simulink model "TosAeb"  (R2021a)
settings: Solver=FixedStepDiscrete  StartTime=0.0  StopTime=inf  FixedStep=0.01  RelTol=1e-3
undefined symbols (not defined in the file or in its folder): ACCModSts, AccSts1, AEB_ObjectMovingOrientation, AEB_ObjectMovingState, CN_BTCActive, CN_CurrentGearR, CN_CurrentGearR_DHT, CN_PTCActive, CN_VDCActive, CN_VSC_EspOff, CN_VSC_FCW_AEB_Error, CN_VSC_FCW_AEB_NoDispay, CN_VSC_FCW_AEB_PerfmDgdtn, CN_VSC_FCW_AEB_TempUnAvlbl, CN_VSC_VehStandstill, CNaccelAvoidMax, CNaccelAvoidMin, CNbrakePedalPress, CNcomfortStrLeftAccelMax, CNcomfortStrLeftAccelMin, CNcomfortStrRightAccelMax, CNcomfortStrRightAccelMin, CNDrvDoorOpen, CNDrvModeEco, CNDrvModeNorm, CNDrvModeOther, CNDrvModeSport, CNDrvSBRreminder, CNNull, CNobjInvalidID, CNPassDoorOpen, CNPassSBRreminder, CNPEBSAccelNegInf, CNPEBSAccelNull, CNPEBSTimeInf, CNPEBSTimeNull, CNSTOPMAX, CNSTOPNULL, CNsubjWidth, CNSwtOFF, CNSwtON, CNTTCMAX, CNTTCNULL, CNVehConfigAEBCycNone, CNVehConfigAEBNone, CNVehConfigAEBPedNone, CNVehConfigFCWNone, CNVehConfigJANone, CollisionSeverity, FALSE, FCWSnvty, JACycObjCalcInfoDefaultParams, JAPedObjCalcInfoDefaultParams, JAVehObjCalcInfoDefaultParams, latCycObjCalcInfoDefaultParams, latPedObjCalcInfoDefaultParams, longObjCalcInfoDefaultParams, ModSts, movingStatus, ObjectType ... (+1112)
libraries: simulink x3031, AEBSFunctionLib x50, Ta_Library x33, AEBSStateMachineLib x23, Mylibrary x12, simulink_extras x7, ObjSelect_lib x3, dspstat3 x2
(part 1 of 102)
settings Model: DataDictionary=ADAS.sldd  Creator=jiapengfei  LastModifiedBy=jiapengfei
settings OptimizationCC: DefaultParameterBehavior=Inlined  EnhancedBackFolding=on  PassReuseOutputArgsAs=Individual arguments  ZeroExternalMemoryAtStartup=off  ZeroInternalMemoryAtStartup=off  OptimizeBlockOrder=speed  DifferentSizesBufferReuse=on  OptimizationCustomize=off  EfficientTunableParamExpr=on
settings DebuggingCC: ParameterTunabilityLossMsg=error
settings SFSimCC: MATLABDynamicMemAlloc=off
settings RTWCC: SystemTargetFile=ert.tlc  GenCodeOnly=on  TemplateMakefile=ert_default_tmf  GenerateReport=on  Toolchain=Microsoft Visual C++ 2019 v16.0 | nmake (64-bit Windows)  BuildConfiguration=Debug  IncludeHyperlinkInReport=on  LaunchReport=on  PortableWordSizes=on  GenerateTraceInfo=on  GenerateTraceReport=on  GenerateTraceReportSl=on  GenerateTraceReportSf=on  GenerateTraceReportEml=on  GenerateCodeMetricsReport=on
settings CodeAppCC: ForceParamTrailComments=on  IgnoreCustomStorageClasses=off  MaxIdLength=128  OperatorAnnotations=on  SimulinkDataObjDesc=on  SFDataObjDesc=on  InsertBlockDesc=on
settings ERTTargetCC: TargetLangStandard=C99 (ISO)  GenerateSampleERTMain=on  InlinedParameterPlacement=NonHierarchical  ERTCustomFileTemplate=example_file_process.tlc  ERTFilePackagingFormat=Compact

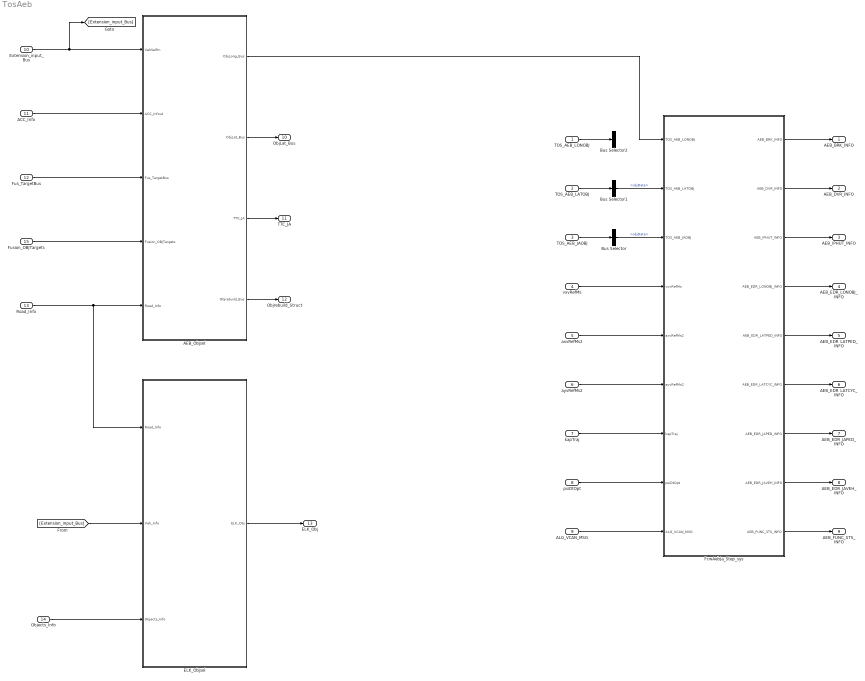
== system TosAeb ==
  TOS_AEB_LONOBJ : Inport  OutDataTypeStr=Bus: ObjectAEBLon_Struct  PortDimensions=1  BusOutputAsStruct=on
  TOS_AEB_LATOBJ : Inport  Port=2  OutDataTypeStr=Bus: ObjectAEBLat_Struct  PortDimensions=1  BusOutputAsStruct=on
  TOS_AEB_JAOBJ : Inport  Port=3  OutDataTypeStr=Bus: Objects_Struct  PortDimensions=1  BusOutputAsStruct=on
  vxvRefMs : Inport  Port=4  OutDataTypeStr=single  PortDimensions=1  BusOutputAsStruct=on
  axvRefMs2 : Inport  Port=5  OutDataTypeStr=single  PortDimensions=1  BusOutputAsStruct=on
  ayvRefMs2 : Inport  Port=6  OutDataTypeStr=single  PortDimensions=1  BusOutputAsStruct=on
  kapTraj : Inport  Port=7  OutDataTypeStr=single  PortDimensions=1  BusOutputAsStruct=on
  psiDtOpt : Inport  Port=8  OutDataTypeStr=single  PortDimensions=1  BusOutputAsStruct=on
  ALG_VCAN_MSG : Inport  Port=9  OutDataTypeStr=Bus: VehicleInfo_Struct  PortDimensions=1  BusOutputAsStruct=on
  Extension_input_Bus : Inport  Port=10  OutDataTypeStr=Bus: Extension_input_Bus  BusOutputAsStruct=on
  ACC_Info : Inport  Port=11  OutDataTypeStr=Bus: ACC_Infout  BusOutputAsStruct=on
  Fus_TargetBus : Inport  Port=12  OutDataTypeStr=Bus: TA_ObjProInfo  BusOutputAsStruct=on
  Road_Info : Inport  Port=13  OutDataTypeStr=Bus: Road_struct  BusOutputAsStruct=on
  Objects_Info : Inport  Port=14  OutDataTypeStr=Bus: Objects_Struct  BusOutputAsStruct=on
  Fusion_OBJTargets : Inport  Port=15  OutDataTypeStr=Bus: Objects_Struct  PortDimensions=1  BusOutputAsStruct=on
  AEB_Objsel : Subsystem
  Bus Selector : BusSelector  OutputSignals=objData
  Bus Selector1 : BusSelector  OutputSignals=objData
  Bus Selector2 : BusSelector  OutputSignals=objData
  ELK_Objsel : Subsystem
  FcwAebJa_Step_sys : Subsystem  TreatAsAtomicUnit=on  SystemSampleTime=0.02
  AEB_BRK_INFO : Outport  OutDataTypeStr=Bus: AEB_BRK_INFO_BUS  PortDimensions=1  BusOutputAsStruct=on
  AEB_DVR_INFO : Outport  Port=2  OutDataTypeStr=Bus: AEB_DVR_INFO_BUS  PortDimensions=1  BusOutputAsStruct=on
  AEB_IPHUT_INFO : Outport  Port=3  OutDataTypeStr=Bus: AEB_IPHUT_INFO_BUS  PortDimensions=1  BusOutputAsStruct=on
  AEB_EDR_LONOBJ_INFO : Outport  Port=4  OutDataTypeStr=Bus: AEB_EDR_LONOBJ_INFO_BUS  PortDimensions=1  BusOutputAsStruct=on
  AEB_EDR_LATPED_INFO : Outport  Port=5  OutDataTypeStr=Bus: AEB_EDR_LATPED_INFO_BUS  PortDimensions=1  BusOutputAsStruct=on
  AEB_EDR_LATCYC_INFO : Outport  Port=6  OutDataTypeStr=Bus: AEB_EDR_LATCYC_INFO_BUS  PortDimensions=1  BusOutputAsStruct=on
  AEB_EDR_JAPED_INFO : Outport  Port=7  OutDataTypeStr=Bus: AEB_EDR_JAPED_INFO_BUS  PortDimensions=1  BusOutputAsStruct=on
  AEB_EDR_JAVEH_INFO : Outport  Port=8  OutDataTypeStr=Bus: AEB_EDR_JAVEH_INFO_BUS  PortDimensions=1  BusOutputAsStruct=on
  AEB_FUNC_STS_INFO : Outport  Port=9  OutDataTypeStr=Bus: AEB_FUNC_STS_INFO_BUS  PortDimensions=1  BusOutputAsStruct=on
  ObjLat_Bus : Outport  Port=10  OutDataTypeStr=Bus: objLat_Bus
  TTC_JA : Outport  Port=11
  Objrebuild_Struct : Outport  Port=12  OutDataTypeStr=Bus: Objrebuild_Bus
  ELK_Obj : Outport  Port=13  OutDataTypeStr=Bus: ObjectELK_Struct  BusOutputAsStruct=on
  wires:
  FcwAebJa_Step_sys/4(AEB_EDR_LONOBJ_INFO) -> AEB_EDR_LONOBJ_INFO/1
  FcwAebJa_Step_sys/7(AEB_EDR_JAPED_INFO) -> AEB_EDR_JAPED_INFO/1
  FcwAebJa_Step_sys/6(AEB_EDR_LATCYC_INFO) -> AEB_EDR_LATCYC_INFO/1
  FcwAebJa_Step_sys/3(AEB_IPHUT_INFO) -> AEB_IPHUT_INFO/1
  FcwAebJa_Step_sys/5(AEB_EDR_LATPED_INFO) -> AEB_EDR_LATPED_INFO/1
  FcwAebJa_Step_sys/2(AEB_DVR_INFO) -> AEB_DVR_INFO/1
  FcwAebJa_Step_sys/1(AEB_BRK_INFO) -> AEB_BRK_INFO/1
  FcwAebJa_Step_sys/9(AEB_FUNC_STS_INFO) -> AEB_FUNC_STS_INFO/1
  FcwAebJa_Step_sys/8(AEB_EDR_JAVEH_INFO) -> AEB_EDR_JAVEH_INFO/1
  TOS_AEB_JAOBJ/1 -> Bus Selector/1
  Bus Selector/1 -> FcwAebJa_Step_sys/3(TOS_AEB_JAOBJ)  "<objData>"
  TOS_AEB_LATOBJ/1 -> Bus Selector1/1
  Bus Selector1/1 -> FcwAebJa_Step_sys/2(TOS_AEB_LATOBJ)  "<objData>"
  TOS_AEB_LONOBJ/1 -> Bus Selector2/1
  psiDtOpt/1 -> FcwAebJa_Step_sys/8(psiDtOpt)
  ayvRefMs2/1 -> FcwAebJa_Step_sys/6(ayvRefMs2)
  kapTraj/1 -> FcwAebJa_Step_sys/7(kapTraj)
  vxvRefMs/1 -> FcwAebJa_Step_sys/4(vxvRefMs)
  axvRefMs2/1 -> FcwAebJa_Step_sys/5(axvRefMs2)
  ALG_VCAN_MSG/1 -> FcwAebJa_Step_sys/9(ALG_VCAN_MSG)
  AEB_Objsel/4(Objrebuild_Bus) -> Objrebuild_Struct/1
  AEB_Objsel/2(ObjLat_Bus) -> ObjLat_Bus/1
  ELK_Objsel/1(ELK_Obj) -> ELK_Obj/1
  AEB_Objsel/3(TTC_JA) -> TTC_JA/1
  Objects_Info/1 -> ELK_Objsel/3(Objects_Info)
  [Extension_input_Bus] -> ELK_Objsel/2(Veh_Info)
  Fus_TargetBus/1 -> AEB_Objsel/3(Fus_TargetBus)
  Road_Info/1 -> ELK_Objsel/1(Road_Info), AEB_Objsel/5(Road_Info)
  ACC_Info/1 -> AEB_Objsel/2(ACC_Infout)
  Extension_input_Bus/1 -> [Extension_input_Bus], AEB_Objsel/1(VehSelfIn)
  AEB_Objsel/1(ObjLong_Bus) -> FcwAebJa_Step_sys/1(TOS_AEB_LONOBJ)
  Fusion_OBJTargets/1 -> AEB_Objsel/4(Fusion_OBJTargets)

== system TosAeb/AEB_Objsel ==
  VehSelfIn : Inport
  ACC_Infout : Inport  Port=2
  Fus_TargetBus : Inport  Port=3
  Fusion_OBJTargets : Inport  Port=4  OutDataTypeStr=Bus: Objects_Struct  PortDimensions=1  BusOutputAsStruct=on
  Road_Info : Inport  Port=5
  Bus Creator : BusCreator  Commented=on  Inputs=3  OutDataTypeStr=Bus: objLat_Bus  NonVirtualBus=on
  Bus Selector1 : BusSelector  OutputSignals=ObjSortNum_Long
  Bus Selector2 : BusSelector  OutputSignals=ID_Lat
  Bus Selector3 : BusSelector  OutputSignals=ObjSortNum_Lat
  Bus Selector4 : BusSelector  OutputSignals=objData
  Constant : Constant
  For Iterator Subsystem : Subsystem  Commented=on  TreatAsAtomicUnit=on
  Lat_Check : MATLAB Function  Commented=on  TreatAsAtomicUnit=on
  Selector : Selector  InputPortWidth=32  IndexOptions=Starting index (port)  Indices=1  OutputSizes=1
  Signal Conversion : SignalConversion  Commented=on  OverrideOpt=off
  Signal Conversion1 : SignalConversion  Commented=on  OverrideOpt=off
  Signal Conversion2 : SignalConversion  Commented=on  OverrideOpt=off
  TA_InfoRebuild : Subsystem
  TA_Processor : Subsystem
  TOS_AEB_Lat_Attributes : Subsystem  TreatAsAtomicUnit=on
  ObjLong_Bus : Outport
  ObjLat_Bus : Outport  Port=2
  TTC_JA : Outport  Port=3
  Objrebuild_Bus : Outport  Port=4
  wires:
  VehSelfIn/1 -> TA_Processor/1(VehSelfIn)
  ACC_Infout/1 -> TA_Processor/2(ACC_Infout)
  TA_Processor/3(TTC_JA) -> TTC_JA/1
  Fus_TargetBus/1 -> TA_InfoRebuild/1(Fus_TargetBus), TA_Processor/3(Fus_TargetBus)
  TA_InfoRebuild/1(Objrebuild_Bus) -> Objrebuild_Bus/1
  Road_Info/1 -> TA_InfoRebuild/2(Road_Info)
  For Iterator Subsystem/1(ttc) -> Lat_Check/3(Lat_TTC)
  Lat_Check/2(Num) -> Signal Conversion/1
  Lat_Check/3(Ttc) -> Signal Conversion2/1
  Signal Conversion2/1 -> Bus Creator/3  "TTC_Lat"
  Signal Conversion/1 -> Bus Creator/2  "ObjSortNum_Lat"
  Bus Selector2/1 -> Lat_Check/1(Lat_ObjID)  "<ID_Lat>"
  Signal Conversion1/1 -> Bus Creator/1  "ID_Lat"
  Lat_Check/1(ID) -> Signal Conversion1/1
  TA_Processor/2(ObjLat_Bus) -> Bus Selector3/1, Bus Selector2/1, ObjLat_Bus/1
  Bus Selector3/1 -> Lat_Check/2(Lat_ObjSortNum), For Iterator Subsystem/1(Lat_ObjSortNum)  "<ObjSortNum_Lat>"
  TA_Processor/1(ObjLong_Bus) -> Bus Selector1/1
  Bus Selector1/1 -> Selector/2  "<ObjSortNum_Long>"
  Fusion_OBJTargets/1 -> Bus Selector4/1
  Bus Selector4/1 -> TOS_AEB_Lat_Attributes/2(TOS_PP_OFM_SignalVerification), For Iterator Subsystem/2(Lat_TTC), Selector/1  "<objData>"
  Selector/1 -> ObjLong_Bus/1
  Constant/1 -> TOS_AEB_Lat_Attributes/1(TOS_AEB_Lat_Index)

== system TosAeb/ELK_Objsel ==
  note: ELK Target Selection
  Road_Info : Inport  OutDataTypeStr=Bus: Road_struct
  Veh_Info : Inport  Port=2
  Objects_Info : Inport  Port=3  OutDataTypeStr=Bus: Objects_Struct
  Bus Creator : BusCreator  Inputs=4  OutDataTypeStr=Bus: ObjectELK_Struct  NonVirtualBus=on
  Bus Selector : BusSelector  OutputSignals=SAS_SteeringWheelSpeed,ABS_VehicleSpeed,SAS_SteeringWheelAngle,YawRate
  Bus Selector2 : BusSelector  OutputSignals=lineData
  Bus Selector3 : BusSelector  OutputSignals=objData
  Bus Selector4 : BusSelector  OutputSignals=timeStamp
  Constant : Constant  Value=1.95  OutDataTypeStr=single
  Constant1 : Constant  Value=0.02
  Constant2 : Constant  Value=3.9  OutDataTypeStr=single
  Constant3 : Constant  Value=4.9  OutDataTypeStr=single
  Constant4 : Constant  Value=0.001  OutDataTypeStr=single
  ELK_Left_Obj_Delay : Subsystem
  ELK_Left_Obj_Delay1 : Subsystem
  ELK_Pre_Cal : Subsystem
  EgoTrajCurvatureForFVSR : Subsystem
  Elk_ObjnumCal : Subsystem
  Info : Subsystem
      mask Display: fprintf('Put your\nimplementation\nhere')
  LanesSelect : Subsystem
  MATLAB Function : MATLAB Function  TreatAsAtomicUnit=on
  Select Object Info1 : Subsystem
  Select Object Info2 : Subsystem
  Signal Conversion{,1..4} (5 alike) : SignalConversion  OverrideOpt=off
  Target_Selection : Subsystem
  Terminator : Terminator
  ELK_Obj : Outport  OutDataTypeStr=Bus: ObjectELK_Struct
  wires:
  [right_boundary_lat_rate_filtered] -> Target_Selection/12(EgoTrajCurvature2)
  Target_Selection/1(dxObj_Vec) -> Terminator/1
  [SteerAngleSpd_degps] -> Target_Selection/8(SteerAngleSpd_degps)
  LanesSelect/1(LeftLineCoef) -> [LDCoef_L]  "LDCoef_L"
  [LDCoef_R] -> ELK_Pre_Cal/3(RightLine)
  Constant1/1 -> [Task_Period]
  [SteerAngle_Deg] -> Target_Selection/7(SteerAngle_Deg)
  ELK_Pre_Cal/2(right_boundary_lat_rate_filtered) -> [right_boundary_lat_rate_filtered]
  ELK_Left_Obj_Delay1/1(ELK_Right_Obj_out) -> [ELK_Right_Obj_out]
  [EgoTrajCurvature] -> Target_Selection/9(EgoTrajCurvature)
  [ELK_Left_Obj] -> ELK_Left_Obj_Delay/1(ELK_Left_Obj)
  [Index_Left_Obj] -> Select Object Info1/2(Index)
  Target_Selection/4(ELK_TA_Right_Flag_Vec) -> [ELK_TA_Right_Flag_Vec]
  [Host_Veh_Width] -> Target_Selection/3(Host_Veh_Width)
  [ELK_Right_Obj] -> ELK_Left_Obj_Delay1/1(ELK_Left_Obj)
  [VehSpdMps] -> EgoTrajCurvatureForFVSR/1(VehSpdMps)
  [ELK_RightLine_Valid_Flag] -> Target_Selection/14(EgoTrajCurvature4)
  ELK_Pre_Cal/1(left_boundary_lat_rate_filtered) -> [left_boundary_lat_rate_filtered]
  [Index_Right_Obj] -> Select Object Info2/2(Index)
  Target_Selection/2(dxObj_abs_Vec) -> [dxObj_abs_Vec]
  [Task_Period] -> ELK_Pre_Cal/4(Task_Period)
  [LDCoef_R] -> Target_Selection/16(LDCoef_R)
  Constant3/1 -> [Host_Veh_Length]
  [Objs_Obstacles] -> Target_Selection/1(Objs_Obstacles)
  [VehSpdMps] -> Target_Selection/5(VehSpdMps)
  [Host_Veh_Length] -> Target_Selection/2(Host_Veh_Length)
  [LDCoef_L] -> ELK_Pre_Cal/2(LeftLine)
  ELK_Pre_Cal/3(ELK_LeftLine_Valid_Flag) -> [ELK_LeftLine_Valid_Flag]
  ELK_Pre_Cal/4(ELK_RightLine_Valid_Flag) -> [ELK_RightLine_Valid_Flag]
  [left_boundary_lat_rate_filtered] -> Target_Selection/11(EgoTrajCurvature1)
  Target_Selection/3(ELK_TA_Left_Flag_Vec) -> [ELK_TA_Left_Flag_Vec]
  [dxDiffObjCoord2VehCoord] -> Target_Selection/4(dxDiffObjCoord2VehCoord)
  MATLAB Function/2(Index_Right_Obj) -> [Index_Right_Obj]  "Meas_Index_Right_Obj"
  [ELK_TA_Left_Flag_Vec] -> MATLAB Function/1(TA_Left_Flag_Vec)
  Select Object Info1/1(TargetInfo) -> [ELK_Left_Obj]
  Constant/1 -> [Host_Veh_Width]
  [Objs_Obstacles] -> Select Object Info1/1(Objs_all)
  LanesSelect/2(RightLineCoef) -> [LDCoef_R]  "LDCoef_R"
  [ELK_TA_Right_Flag_Vec] -> MATLAB Function/2(TA_Right_Flag_Vec)
  [Objs_Obstacles] -> Select Object Info2/1(Objs_all)
  ELK_Left_Obj_Delay/1(ELK_Left_Obj_out) -> [ELK_Left_Obj_out]
  Select Object Info2/1(TargetInfo) -> [ELK_Right_Obj]
  [LDCoef_L] -> Target_Selection/15(LDCoef_L)
  [dxObj_abs_Vec] -> MATLAB Function/3(dx_Obj_vector)
  EgoTrajCurvatureForFVSR/1(EgoTrajCurvatureForFVSR) -> [EgoTrajCurvatureForFVSR]
  [ELK_LeftLine_Valid_Flag] -> Target_Selection/13(EgoTrajCurvature3)
  [YawRate_Degps] -> Target_Selection/6(YawRate_Degps)
  [EgoTrajCurvature] -> EgoTrajCurvatureForFVSR/2(EgoTrajCurvature)
  [EgoTrajCurvatureForFVSR] -> Target_Selection/10(EgoTrajCurvatureForFVSR)
  MATLAB Function/1(Index_Left_Obj) -> [Index_Left_Obj]  "Meas_Index_Left_Obj"
  [Host_Veh_Width] -> ELK_Pre_Cal/1(Host_Veh_Width)
  Constant2/1 -> [dxDiffObjCoord2VehCoord]
  Veh_Info/1 -> Bus Selector/1
  Bus Selector/1 -> Signal Conversion3/1  "<SAS_SteeringWheelSpeed>"
  Bus Selector/2 -> Signal Conversion2/1  "<ABS_VehicleSpeed>"
  Bus Selector/3 -> Signal Conversion1/1  "<SAS_SteeringWheelAngle>"
  Bus Selector/4 -> Signal Conversion/1  "<YawRate>"
  Signal Conversion/1 -> [YawRate_Degps]
  Signal Conversion1/1 -> [SteerAngle_Deg]
  Signal Conversion2/1 -> [VehSpdMps]
  Signal Conversion3/1 -> [SteerAngleSpd_degps]
  Constant4/1 -> Signal Conversion4/1
  Signal Conversion4/1 -> [EgoTrajCurvature]
  Road_Info/1 -> Bus Selector2/1
  Bus Selector2/1 -> LanesSelect/1(lineData)  "<lineData>"
  Objects_Info/1 -> [allObject], Bus Selector3/1
  Bus Selector3/1 -> [Objs_Obstacles]  "<objData>"
  [ELK_Left_Obj_out] -> Elk_ObjnumCal/1(Left)
  [ELK_Right_Obj_out] -> Elk_ObjnumCal/2(Right)
  [allObject] -> Bus Selector4/1
  Bus Selector4/1 -> Bus Creator/1  "<timeStamp>"
  Elk_ObjnumCal/1(objnum) -> Bus Creator/2  "objNum"
  [ELK_Left_Obj_out] -> Bus Creator/3  "objELKLeft"
  [ELK_Right_Obj_out] -> Bus Creator/4  "objELKRight"
  Bus Creator/1 -> ELK_Obj/1

== system TosAeb/FcwAebJa_Step_sys ==
  TOS_AEB_LONOBJ : Inport  OutDataTypeStr=Bus: Object_Struct  PortDimensions=1  BusOutputAsStruct=on
  TOS_AEB_LATOBJ : Inport  Port=2  OutDataTypeStr=Bus: Object_Struct  PortDimensions=10  BusOutputAsStruct=on
  TOS_AEB_JAOBJ : Inport  Port=3  OutDataTypeStr=Bus: Object_Struct  PortDimensions=32  BusOutputAsStruct=on
  vxvRefMs : Inport  Port=4  OutDataTypeStr=single  PortDimensions=1  BusOutputAsStruct=on
  axvRefMs2 : Inport  Port=5  OutDataTypeStr=single  PortDimensions=1  BusOutputAsStruct=on
  ayvRefMs2 : Inport  Port=6  OutDataTypeStr=single  PortDimensions=1  BusOutputAsStruct=on
  kapTraj : Inport  Port=7  OutDataTypeStr=single  PortDimensions=1  BusOutputAsStruct=on
  psiDtOpt : Inport  Port=8  OutDataTypeStr=single  PortDimensions=1  BusOutputAsStruct=on
  ALG_VCAN_MSG : Inport  Port=9  OutDataTypeStr=Bus: VehicleInfo_Struct  PortDimensions=1  BusOutputAsStruct=on
  ALG_AEBSystem : Subsystem  TreatAsAtomicUnit=on
  AEB_BRK_INFO : Outport  OutDataTypeStr=Bus: AEB_BRK_INFO_BUS  PortDimensions=1  BusOutputAsStruct=on
  AEB_DVR_INFO : Outport  Port=2  OutDataTypeStr=Bus: AEB_DVR_INFO_BUS  PortDimensions=1  BusOutputAsStruct=on
  AEB_IPHUT_INFO : Outport  Port=3  OutDataTypeStr=Bus: AEB_IPHUT_INFO_BUS  PortDimensions=1  BusOutputAsStruct=on
  AEB_EDR_LONOBJ_INFO : Outport  Port=4  OutDataTypeStr=Bus: AEB_EDR_LONOBJ_INFO_BUS  PortDimensions=1  BusOutputAsStruct=on
  AEB_EDR_LATPED_INFO : Outport  Port=5  OutDataTypeStr=Bus: AEB_EDR_LATPED_INFO_BUS  PortDimensions=1  BusOutputAsStruct=on
  AEB_EDR_LATCYC_INFO : Outport  Port=6  OutDataTypeStr=Bus: AEB_EDR_LATCYC_INFO_BUS  PortDimensions=1  BusOutputAsStruct=on
  AEB_EDR_JAPED_INFO : Outport  Port=7  OutDataTypeStr=Bus: AEB_EDR_JAPED_INFO_BUS  PortDimensions=1  BusOutputAsStruct=on
  AEB_EDR_JAVEH_INFO : Outport  Port=8  OutDataTypeStr=Bus: AEB_EDR_JAVEH_INFO_BUS  PortDimensions=1  BusOutputAsStruct=on
  AEB_FUNC_STS_INFO : Outport  Port=9  OutDataTypeStr=Bus: AEB_FUNC_STS_INFO_BUS  PortDimensions=1  BusOutputAsStruct=on
  wires:
  ALG_AEBSystem/4(AEB_EDR_LONOBJ_INFO) -> AEB_EDR_LONOBJ_INFO/1
  ALG_AEBSystem/9(AEB_FUNC_STS_INFO) -> AEB_FUNC_STS_INFO/1
  ALG_AEBSystem/8(AEB_EDR_JAVEH_INFO) -> AEB_EDR_JAVEH_INFO/1
  ALG_AEBSystem/2(AEB_DVR_INFO) -> AEB_DVR_INFO/1
  ALG_AEBSystem/3(AEB_IPHUT_INFO) -> AEB_IPHUT_INFO/1
  ALG_AEBSystem/7(AEB_EDR_JAPED_INFO) -> AEB_EDR_JAPED_INFO/1
  ALG_AEBSystem/1(AEB_BRK_INFO) -> AEB_BRK_INFO/1
  ALG_AEBSystem/5(AEB_EDR_LATPED_INFO) -> AEB_EDR_LATPED_INFO/1
  ALG_AEBSystem/6(AEB_EDR_LATCYC_INFO) -> AEB_EDR_LATCYC_INFO/1
  TOS_AEB_LONOBJ/1 -> ALG_AEBSystem/1(TOS_AEB_LONOBJ)
  TOS_AEB_LATOBJ/1 -> ALG_AEBSystem/2(TOS_AEB_LATOBJ)
  TOS_AEB_JAOBJ/1 -> ALG_AEBSystem/3(TOS_AEB_JAOBJ)
  vxvRefMs/1 -> ALG_AEBSystem/4(vxvRefMs)
  axvRefMs2/1 -> ALG_AEBSystem/5(axvRefMs2)
  ayvRefMs2/1 -> ALG_AEBSystem/6(ayvRefMs2)
  kapTraj/1 -> ALG_AEBSystem/7(kapTraj)
  psiDtOpt/1 -> ALG_AEBSystem/8(psiDtOpt)
  ALG_VCAN_MSG/1 -> ALG_AEBSystem/9(ALG_VCAN_MSG)

== system TosAeb/AEB_Objsel/For Iterator Subsystem ==
  Lat_ObjSortNum : Inport
  Lat_TTC : Inport  Port=2
  Assignment : Assignment  DiagnosticForDimensions=Warning  IndexOptions=Index vector (port)  Indices=1  OutputSizes=1
  Compare To Constant : simulink/Logic and Bit Operations/Compare To Constant  relop===  const=0  OutDataTypeStr=boolean
  Constant : Constant  Value=zeros(10,1)  OutDataTypeStr=single
  Constant1 : Constant  Value=100  OutDataTypeStr=single
  Constant2 : Constant  OutDataTypeStr=uint8
  For Iterator : ForIterator  IterationLimit=10
  Index Vector : MultiPortSwitch  Inputs=1
  Index Vector1 : MultiPortSwitch  Inputs=1
  Switch : Switch  Criteria=u2 > Threshold
  Switch1 : Switch  Criteria=u2 > Threshold
  ttc : Outport
  wires:
  Constant/1 -> Assignment/1
  Assignment/1 -> ttc/1
  For Iterator/1 -> [Index]
  [Index] -> Assignment/3
  [Index] -> Index Vector1/1
  Lat_ObjSortNum/1 -> Index Vector1/2
  Index Vector1/1 -> Switch1/3, Compare To Constant/1
  Compare To Constant/1 -> Switch1/2, Switch/2
  Constant1/1 -> Switch/1
  Constant2/1 -> Switch1/1
  Switch1/1 -> Index Vector/1
  Lat_TTC/1 -> Index Vector/2
  Switch/1 -> Assignment/2
  Index Vector/1 -> Switch/3

== system TosAeb/AEB_Objsel/TA_InfoRebuild ==
  Fus_TargetBus : Inport
  Road_Info : Inport  Port=2
  TAObjInfoCalc : Subsystem  TreatAsAtomicUnit=on
  Objrebuild_Bus : Outport
  wires:
  Fus_TargetBus/1 -> TAObjInfoCalc/1(Fus_TargetBus)
  Road_Info/1 -> TAObjInfoCalc/2(Road_Info)
  TAObjInfoCalc/1(Objrebuild_Struct) -> Objrebuild_Bus/1

== system TosAeb/AEB_Objsel/TA_Processor ==
  VehSelfIn : Inport
  ACC_Infout : Inport  Port=2
  Fus_TargetBus : Inport  Port=3
  AEB_ObjSelect : Subsystem
  PreProcessor : Subsystem
  TA_Check : Subsystem
  ObjLong_Bus : Outport
  ObjLat_Bus : Outport  Port=2
  TTC_JA : Outport  Port=3
  wires:
  VehSelfIn/1 -> PreProcessor/1(VehSelfIn)  "VehSelf"
  Fus_TargetBus/1 -> PreProcessor/3(Fus_TargetBus)
  PreProcessor/4(AccTgtIdn) -> AEB_ObjSelect/4(AccTgtIdn)
  PreProcessor/1(VehSelfData) -> AEB_ObjSelect/1(VehSelfData)
  ACC_Infout/1 -> PreProcessor/2(ACC_Infout)
  AEB_ObjSelect/1(Long_ObjSortNum) -> TA_Check/1(Long_ObjSortNum)
  PreProcessor/2(TA_ObjSumInfo) -> AEB_ObjSelect/2(TA_ObjSumInfo)
  PreProcessor/3(PathEstimn) -> AEB_ObjSelect/3(PathEstimn)
  AEB_ObjSelect/7(Lat_TTC) -> TA_Check/7(Lat_TTC)
  AEB_ObjSelect/2(Long_ObjID) -> TA_Check/2(Long_ObjID)
  AEB_ObjSelect/5(Ta_Info) -> TA_Check/5(Ta_Info)
  AEB_ObjSelect/3(Lat_ObjSortNum) -> TA_Check/3(Lat_ObjSortNum)
  AEB_ObjSelect/4(Lat_ObjID) -> TA_Check/4(Lat_ObjID)
  AEB_ObjSelect/6(Long_TTC) -> TA_Check/6(Long_TTC)
  AEB_ObjSelect/8(JA_TTC) -> TA_Check/8(JA_TTC)
  TA_Check/1(ObjLong_Bus) -> ObjLong_Bus/1
  TA_Check/2(ObjLat_Bus) -> ObjLat_Bus/1
  TA_Check/3(TTC_JA) -> TTC_JA/1

== system TosAeb/AEB_Objsel/TOS_AEB_Lat_Attributes ==
  TOS_AEB_Lat_Index : Inport  Partition=on  PartitionDimension=1  PartitionWidth=1  PartitionOffset=0
  TOS_PP_OFM_SignalVerification : Inport  Port=2
  Compare To Constant : simulink/Logic and Bit Operations/Compare To Constant  relop=~=  const=0  OutDataTypeStr=boolean
  For Each : ForEach  DisableCoverage=on
  If : If  IfExpression=u1 == 0
  If Action Subsystem : Subsystem  TreatAsAtomicUnit=on
  If Action Subsystem1 : Subsystem  TreatAsAtomicUnit=on
  Merge : Merge
  wires:
  TOS_AEB_Lat_Index/1 -> [TOS_AEB_Lat_Index], Compare To Constant/1
  Compare To Constant/1 -> If/1
  If/1 -> If Action Subsystem/Action
  TOS_PP_OFM_SignalVerification/1 -> If Action Subsystem1/2(TOS_PP_OFM_SignalVerification)
  If/2 -> If Action Subsystem1/Action
  [TOS_AEB_Lat_Index] -> If Action Subsystem1/1(TOS_AEB_Lat_Index)
  If Action Subsystem/1(Out1) -> Merge/1
  If Action Subsystem1/1(Out1) -> Merge/2

== system TosAeb/ELK_Objsel/ELK_Left_Obj_Delay ==
  ELK_Left_Obj : Inport
  Bus Creator : BusCreator  Commented=on  Inputs=3  OutDataTypeStr=Bus: ObjectELK_Struct  NonVirtualBus=on
  Bus Selector2 : BusSelector  OutputSignals=objID
  Compare To Constant : simulink/Logic and Bit Operations/Compare To Constant  Commented=on  relop===  const=0  OutDataTypeStr=boolean
  Constant : Constant  Commented=on  Value=50
  Constant1 : Constant  Commented=on  Value=0
  Constant10 : Constant  Value=0
  Constant11 : Constant  Value=0
  Constant2 : Constant  Commented=on  Value=0
  Constant3 : Constant  Commented=on  OutDataTypeStr=int64_t
  Constant4 : Constant  Commented=on  OutDataTypeStr=uint8_t
  Constant5 : Constant  Commented=on  Value=0  OutDataTypeStr=uint8_t
  Constant9 : Constant  Value=0
  From1 : From  Commented=on  GotoTag=ELK_Left_Obj_ID
  From51 : From  Commented=on  GotoTag=ELK_Left_Obj_ID
  Hysteresis2 : Stateflow chart  Commented=on  TreatAsAtomicUnit=on
  Relational Operator : RelationalOperator  Commented=on  Operator=~=  OutDataTypeStr=boolean  RndMeth=Simplest
  Relational Operator10 : RelationalOperator  Operator=>  OutDataTypeStr=boolean  RndMeth=Simplest
  Relational Operator7 : RelationalOperator  Commented=on  Operator===  OutDataTypeStr=boolean  RndMeth=Simplest
  Relational Operator8 : RelationalOperator  Operator=>  OutDataTypeStr=boolean  RndMeth=Simplest
  Relational Operator9 : RelationalOperator  Operator=>  OutDataTypeStr=boolean  RndMeth=Simplest
  Switch{,1..3} (4 alike) : Switch  Criteria=u2 > Threshold
  Switch4 : Switch  Commented=on  Criteria=u2 > Threshold
  Trigger Hold1 : Subsystem  Commented=on
  Unit Delay{,1,4..7} (6 alike) : UnitDelay  SampleTime=-1  HasFrameUpgradeWarning=on
  ELK_Left_Obj_out : Outport  OutDataTypeStr=Bus: Object_Struct
  wires:
  Trigger Hold1/1(Out) -> ?
  Unit Delay1/1 -> [ELK_Left_Obj_K1], Unit Delay4/1
  Unit Delay/1 -> Unit Delay6/1, [ELK_Left_Obj_ID_K1]
  [ELK_Left_Obj] -> Unit Delay1/1
  [ELK_Left_Obj_ID_K1] -> Relational Operator9/1
  Constant10/1 -> Relational Operator9/2
  Switch/1 -> [ELK_Left_Obj_out]
  [ELK_Left_Obj] -> Switch/1
  Constant2/1 -> Relational Operator7/2
  Hysteresis2/1(Flag) -> Trigger Hold1/1(Trigger)
  Constant/1 -> Trigger Hold1/2(hold_time)
  Relational Operator/1 -> Hysteresis2/1(Input_1)
  From51/1 -> Relational Operator7/1, Relational Operator/1
  Relational Operator7/1 -> Hysteresis2/2(Input_2)
  [ELK_Left_Obj] -> Bus Selector2/1
  Constant1/1 -> Relational Operator/2
  [ELK_Left_Obj_ID] -> Switch/2
  Unit Delay4/1 -> Unit Delay5/1, [ELK_Left_Obj_K2]
  Relational Operator9/1 -> Switch1/2
  Unit Delay7/1 -> [ELK_Left_Obj_ID_K3]
  Unit Delay5/1 -> [ELK_Left_Obj_K3]
  Unit Delay6/1 -> Unit Delay7/1, [ELK_Left_Obj_ID_K2]
  Switch2/1 -> Switch1/3
  Switch1/1 -> Switch/3
  [ELK_Left_Obj_K2] -> Switch2/1
  [ELK_Left_Obj] -> Switch3/3
  [ELK_Left_Obj_K1] -> Switch1/1
  [ELK_Left_Obj_ID_K2] -> Relational Operator8/1
  Constant9/1 -> Relational Operator8/2
  Relational Operator8/1 -> Switch2/2
  [ELK_Left_Obj_K3] -> Switch3/1
  [ELK_Left_Obj_ID_K3] -> Relational Operator10/1
  Constant11/1 -> Relational Operator10/2
  Relational Operator10/1 -> Switch3/2
  Switch3/1 -> Switch2/3
  Bus Selector2/1 -> Unit Delay/1, [ELK_Left_Obj_ID]  "<objID>"
  ELK_Left_Obj/1 -> [ELK_Left_Obj]
  From1/1 -> Compare To Constant/1
  Compare To Constant/1 -> Switch4/2
  Constant4/1 -> Switch4/1
  Constant5/1 -> Switch4/3
  Constant3/1 -> Bus Creator/1  "timeStamp"
  Switch4/1 -> Bus Creator/2  "objNum"
  Bus Creator/1 -> ?
  [ELK_Left_Obj_out] -> ELK_Left_Obj_out/1  "objData"

== system TosAeb/ELK_Objsel/ELK_Left_Obj_Delay1 ==
  ELK_Left_Obj : Inport
  Bus Creator : BusCreator  Commented=on  Inputs=3  OutDataTypeStr=Bus: ObjectELK_Struct  NonVirtualBus=on
  Bus Selector2 : BusSelector  OutputSignals=objID
  Compare To Constant : simulink/Logic and Bit Operations/Compare To Constant  Commented=on  relop===  const=0  OutDataTypeStr=boolean
  Constant : Constant  Commented=on  Value=50
  Constant1 : Constant  Commented=on  Value=0
  Constant10 : Constant  Value=0
  Constant11 : Constant  Value=0
  Constant2 : Constant  Commented=on  Value=0
  Constant3 : Constant  Commented=on  OutDataTypeStr=int64_t
  Constant4 : Constant  Commented=on  OutDataTypeStr=uint8_t
  Constant5 : Constant  Commented=on  Value=0  OutDataTypeStr=uint8_t
  Constant9 : Constant  Value=0
  From2 : From  Commented=on  GotoTag=ELK_Left_Obj_ID
  From51 : From  Commented=on  GotoTag=ELK_Left_Obj_ID
  Hysteresis2 : Stateflow chart  Commented=on  TreatAsAtomicUnit=on
  Relational Operator : RelationalOperator  Commented=on  Operator=~=  OutDataTypeStr=boolean  RndMeth=Simplest
  Relational Operator10 : RelationalOperator  Operator=>  OutDataTypeStr=boolean  RndMeth=Simplest
  Relational Operator7 : RelationalOperator  Commented=on  Operator===  OutDataTypeStr=boolean  RndMeth=Simplest
  Relational Operator8 : RelationalOperator  Operator=>  OutDataTypeStr=boolean  RndMeth=Simplest
  Relational Operator9 : RelationalOperator  Operator=>  OutDataTypeStr=boolean  RndMeth=Simplest
  Switch{,1..3} (4 alike) : Switch  Criteria=u2 > Threshold
  Switch4 : Switch  Commented=on  Criteria=u2 > Threshold
  Trigger Hold1 : Subsystem  Commented=on
  Unit Delay{,1,4..7} (6 alike) : UnitDelay  SampleTime=-1  HasFrameUpgradeWarning=on
  ELK_Right_Obj_out : Outport  OutDataTypeStr=Bus: Object_Struct
  wires:
  [ELK_Left_Obj_out] -> ELK_Right_Obj_out/1
  ELK_Left_Obj/1 -> [ELK_Left_Obj]
  Bus Selector2/1 -> [ELK_Left_Obj_ID], Unit Delay/1  "<objID>"
  Switch3/1 -> Switch2/3
  Relational Operator10/1 -> Switch3/2
  Constant11/1 -> Relational Operator10/2
  [ELK_Left_Obj_ID_K3] -> Relational Operator10/1
  [ELK_Left_Obj_K3] -> Switch3/1
  Relational Operator8/1 -> Switch2/2
  Constant9/1 -> Relational Operator8/2
  [ELK_Left_Obj_ID_K2] -> Relational Operator8/1
  [ELK_Left_Obj_K1] -> Switch1/1
  [ELK_Left_Obj] -> Switch3/3
  [ELK_Left_Obj_K2] -> Switch2/1
  Switch1/1 -> Switch/3
  Switch2/1 -> Switch1/3
  Unit Delay6/1 -> [ELK_Left_Obj_ID_K2], Unit Delay7/1
  Unit Delay5/1 -> [ELK_Left_Obj_K3]
  Unit Delay7/1 -> [ELK_Left_Obj_ID_K3]
  Relational Operator9/1 -> Switch1/2
  Unit Delay4/1 -> [ELK_Left_Obj_K2], Unit Delay5/1
  [ELK_Left_Obj_ID] -> Switch/2
  Constant1/1 -> Relational Operator/2
  [ELK_Left_Obj] -> Bus Selector2/1
  Relational Operator7/1 -> Hysteresis2/2(Input_2)
  From51/1 -> Relational Operator/1, Relational Operator7/1
  Relational Operator/1 -> Hysteresis2/1(Input_1)
  Constant/1 -> Trigger Hold1/2(hold_time)
  Hysteresis2/1(Flag) -> Trigger Hold1/1(Trigger)
  Constant2/1 -> Relational Operator7/2
  [ELK_Left_Obj] -> Switch/1
  Switch/1 -> [ELK_Left_Obj_out]
  Constant10/1 -> Relational Operator9/2
  [ELK_Left_Obj_ID_K1] -> Relational Operator9/1
  [ELK_Left_Obj] -> Unit Delay1/1
  Unit Delay/1 -> [ELK_Left_Obj_ID_K1], Unit Delay6/1
  Unit Delay1/1 -> Unit Delay4/1, [ELK_Left_Obj_K1]
  Trigger Hold1/1(Out) -> ?
  Switch4/1 -> Bus Creator/2  "objNum"
  Constant4/1 -> Switch4/1
  Constant5/1 -> Switch4/3
  Compare To Constant/1 -> Switch4/2
  Constant3/1 -> Bus Creator/1  "timeStamp"
  From2/1 -> Compare To Constant/1

== system TosAeb/ELK_Objsel/ELK_Pre_Cal ==
  note: 以车道线的常数项作为自车当前与车道线的距离并不是自车到车道线的垂直距离
      | 同样通过微分求得的自车朝车道线运动的横向速度并不是垂直于车道线
      | 可根据车道线在自车位置处的曲率来进行分解
  Host_Veh_Width : Inport
  LeftLine : Inport  Port=2
  RightLine : Inport  Port=3
  Task_Period : Inport  Port=4
  Add{3,5..6,9} (4 alike) : Sum  Inputs=+-
  Bus Selector : BusSelector  OutputSignals=LineQuality,LineWidth,LineA0
  Bus Selector1 : BusSelector  OutputSignals=LineQuality,LineWidth,LineA0
  Constant1 : Constant  Description=_GWMH9P5.ELK.000_27_
  Constant10 : Constant  Description=_GWMH9P5.ELK.000_27_  Value=0.1
  Constant2 : Constant  Description=_GWMH9P5.ELK.000_27_
  Constant3 : Constant  Value=3
  Constant4 : Constant  Value=2
  Constant5 : Constant  Value=2
  Constant6 : Constant  Value=3
  Constant66 : Constant  Value=0.5
  Constant68 : Constant  Value=0.1
  Constant69 : Constant  Value=0.5
  Constant7 : Constant
  Constant70 : Constant  Value=0.1
  Constant8 : Constant
  Constant9 : Constant  Description=_GWMH9P5.ELK.000_27_  Value=0.1
  Derivative : Derivative  Commented=on
  Derivative1 : Derivative  Commented=on
  Divide : Product  Inputs=*/
  Divide2 : Product  Inputs=*/
  First_Order_Lag_Filter : ObjSelect_lib/First_Order_Lag_Filter  SystemSampleTime=-1  VariantActivationTime=update diagram  Latency=0  AutoFrameSizeCalculation=off
  First_Order_Lag_Filter1 : ObjSelect_lib/First_Order_Lag_Filter  SystemSampleTime=-1  VariantActivationTime=update diagram  Latency=0  AutoFrameSizeCalculation=off
  From23 : From  Commented=on  GotoTag=curr_left_dis_to_mkr
  From44 : From  Commented=on  GotoTag=curr_right_dis_to_mkr
  Gain11 : Gain  Gain=-1
  Gain3 : Gain  Gain=0.5
  Gain4 : Gain  Gain=0.5
  Goto14 : Goto  Commented=on  GotoTag=right_boundary_lat_rate
  Goto43 : Goto  Commented=on  GotoTag=left_boundary_lat_rate
  Logical Operator{4,6,9..10} (4 alike) : Logic  AllPortsSameDT=off  OutDataTypeStr=boolean
  Logical Operator{2,5,7..8} (4 alike) : Logic  Operator=OR  AllPortsSameDT=off  OutDataTypeStr=boolean
  Relational Operator{1..6} (6 alike) : RelationalOperator  Operator===  OutDataTypeStr=boolean  RndMeth=Nearest
  Relational Operator10 : RelationalOperator  OutDataTypeStr=boolean  RndMeth=Simplest
  Relational Operator7 : RelationalOperator  Operator=<=  OutDataTypeStr=boolean  RndMeth=Simplest
  Relational Operator8 : RelationalOperator  OutDataTypeStr=boolean  RndMeth=Simplest
  Relational Operator9 : RelationalOperator  Operator=<=  OutDataTypeStr=boolean  RndMeth=Simplest
  Unit Delay2 : UnitDelay  SampleTime=-1  HasFrameUpgradeWarning=on
  Unit Delay3 : UnitDelay  SampleTime=-1  HasFrameUpgradeWarning=on
  left_boundary_lat_rate_filtered : Outport
  right_boundary_lat_rate_filtered : Outport  Port=2
  ELK_LeftLine_Valid_Flag : Outport  Port=3
  ELK_RightLine_Valid_Flag : Outport  Port=4
  wires:
  Host_Veh_Width/1 -> [Host_Veh_Width]
  LeftLine/1 -> Bus Selector/1
  Bus Selector/3 -> [a0_LeftLine]  "<LineA0>"
  Bus Selector/2 -> [left_line_width]  "<LineWidth>"
  RightLine/1 -> Bus Selector1/1
  Bus Selector1/2 -> [right_line_width]  "<LineWidth>"
  Bus Selector1/3 -> [a0_RightLine]  "<LineA0>"
  Constant4/1 -> Relational Operator4/2
  Relational Operator4/1 -> Logical Operator5/1
  Constant6/1 -> Relational Operator6/2
  Relational Operator6/1 -> Logical Operator5/2
  Logical Operator5/1 -> Logical Operator8/1
  [Le_Confidence] -> Relational Operator4/1, Relational Operator2/1, Relational Operator6/1
  Constant8/1 -> Relational Operator2/2
  Relational Operator2/1 -> Logical Operator8/2
  Bus Selector/1 -> [Le_Confidence]  "<LineQuality>"
  Bus Selector1/1 -> [Ri_Confidence]  "<LineQuality>"
  Logical Operator7/1 -> [Ri_Valid_Flag]
  Constant3/1 -> Relational Operator3/2
  Relational Operator3/1 -> Logical Operator2/2
  Constant5/1 -> Relational Operator5/2
  Relational Operator5/1 -> Logical Operator2/1
  Logical Operator2/1 -> Logical Operator7/2
  [Ri_Confidence] -> Relational Operator1/1, Relational Operator5/1, Relational Operator3/1
  Constant7/1 -> Relational Operator1/2
  Relational Operator1/1 -> Logical Operator7/1
  Logical Operator8/1 -> [Le_Valid_Flag]
  Task_Period/1 -> [Task_Period]
  [a0_LeftLine] -> Add6/1
  Add6/1 -> [curr_left_dis_to_mkr]
  [Host_Veh_Width] -> Gain3/1
  Gain3/1 -> Add6/2
  From23/1 -> Derivative/1
  Derivative/1 -> Goto43/1
  [curr_left_dis_to_mkr] -> Add9/1, Unit Delay2/1
  Unit Delay2/1 -> Add9/2
  Add9/1 -> Divide/1
  Divide/1 -> [left_boundary_lat_rate]
  [Task_Period] -> Divide/2
  Add3/1 -> [curr_right_dis_to_mkr]
  [Host_Veh_Width] -> Gain4/1
  Gain4/1 -> Add3/2
  From44/1 -> Derivative1/1
  Derivative1/1 -> Goto14/1
  [a0_RightLine] -> Gain11/1
  Gain11/1 -> Add3/1
  [curr_right_dis_to_mkr] -> Add5/1, Unit Delay3/1
  Unit Delay3/1 -> Add5/2
  Add5/1 -> Divide2/1
  Divide2/1 -> [right_boundary_lat_rate]
  [Task_Period] -> Divide2/2
  [Le_Valid_Flag] -> Logical Operator9/1
  [left_line_width] -> Relational Operator8/1, Relational Operator7/1
  Logical Operator10/1 -> Logical Operator9/2
  Relational Operator8/1 -> Logical Operator10/2
  Relational Operator7/1 -> Logical Operator10/1
  Logical Operator9/1 -> [ELK_LeftLine_Valid_Flag]
  [Ri_Valid_Flag] -> Logical Operator4/1
  Logical Operator4/1 -> [ELK_RightLine_Valid_Flag]
  Constant66/1 -> Relational Operator7/2
  Constant68/1 -> Relational Operator8/2
  [right_line_width] -> Relational Operator10/1, Relational Operator9/1
  Logical Operator6/1 -> Logical Operator4/2
  Relational Operator10/1 -> Logical Operator6/2
  Relational Operator9/1 -> Logical Operator6/1
  Constant69/1 -> Relational Operator9/2
  Constant70/1 -> Relational Operator10/2
  [left_boundary_lat_rate] -> First_Order_Lag_Filter1/3
  Constant1/1 -> First_Order_Lag_Filter1/1
  Constant9/1 -> First_Order_Lag_Filter1/2
  First_Order_Lag_Filter1/1 -> [left_boundary_lat_rate_filtered]
  [right_boundary_lat_rate] -> First_Order_Lag_Filter/3
  Constant2/1 -> First_Order_Lag_Filter/1
  Constant10/1 -> First_Order_Lag_Filter/2
  First_Order_Lag_Filter/1 -> [right_boundary_lat_rate_filtered]
  [left_boundary_lat_rate_filtered] -> left_boundary_lat_rate_filtered/1
  [right_boundary_lat_rate_filtered] -> right_boundary_lat_rate_filtered/1
  [ELK_LeftLine_Valid_Flag] -> ELK_LeftLine_Valid_Flag/1
  [ELK_RightLine_Valid_Flag] -> ELK_RightLine_Valid_Flag/1

== system TosAeb/ELK_Objsel/EgoTrajCurvatureForFVSR ==
  VehSpdMps : Inport
  EgoTrajCurvature : Inport  Port=2
  Constant4 : Constant  Value=ObjSelect_CalFVSREgoSpdStandstill
  Relational Operator : RelationalOperator  Operator=<=  OutDataTypeStr=boolean  RndMeth=Simplest
  Switch : Switch  Criteria=u2 > Threshold
  Unit Delay : UnitDelay  SampleTime=-1  HasFrameUpgradeWarning=on
  EgoTrajCurvatureForFVSR : Outport
  wires:
  Relational Operator/1 -> Switch/2
  [EgoTrajCurvatureK1] -> Switch/1
  Unit Delay/1 -> [EgoTrajCurvatureK1]
  Constant4/1 -> Relational Operator/2
  [VehSpdMps] -> Relational Operator/1
  [EgoTrajCurvature] -> Switch/3
  Switch/1 -> Unit Delay/1, [EgoTrajCurvatureForFVSR]
  VehSpdMps/1 -> [VehSpdMps]
  EgoTrajCurvature/1 -> [EgoTrajCurvature]
  [EgoTrajCurvatureForFVSR] -> EgoTrajCurvatureForFVSR/1

== system TosAeb/ELK_Objsel/Elk_ObjnumCal ==
  Left : Inport
  Right : Inport  Port=2
  Add : Sum
  Bus Selector4 : BusSelector  OutputSignals=objID
  Bus Selector5 : BusSelector  OutputSignals=objID
  Compare To Constant : simulink/Logic and Bit Operations/Compare To Constant  relop=~=  const=0  OutDataTypeStr=boolean
  Compare To Constant1 : simulink/Logic and Bit Operations/Compare To Constant  relop=~=  const=0  OutDataTypeStr=boolean
  Constant5 : Constant  OutDataTypeStr=uint8_t
  Constant6 : Constant  Value=0  OutDataTypeStr=uint8_t
  Constant7 : Constant  OutDataTypeStr=uint8_t
  Constant8 : Constant  Value=0  OutDataTypeStr=uint8_t
  Switch : Switch  Criteria=u2 > Threshold
  Switch1 : Switch  Criteria=u2 > Threshold
  objnum : Outport
  wires:
  Switch1/1 -> Add/2
  Bus Selector4/1 -> Compare To Constant/1  "<objID>"
  Constant5/1 -> Switch/1
  Add/1 -> objnum/1
  Constant7/1 -> Switch1/1
  Compare To Constant1/1 -> Switch1/2
  Compare To Constant/1 -> Switch/2
  Switch/1 -> Add/1
  Constant8/1 -> Switch1/3
  Bus Selector5/1 -> Compare To Constant1/1  "<objID>"
  Constant6/1 -> Switch/3
  Left/1 -> Bus Selector4/1
  Right/1 -> Bus Selector5/1

== system TosAeb/ELK_Objsel/Info ==

== system TosAeb/ELK_Objsel/LanesSelect ==
  note: 0 left
      | 1 right
  lineData : Inport  OutDataTypeStr=Bus: LineData_Struct
  Bus Creator2 : BusCreator  Inputs=10
  Bus Creator3 : BusCreator  Inputs=10
  Bus Selector14 : BusSelector  OutputSignals=lineSegs
  Bus Selector15 : BusSelector  OutputSignals=lineSegs
  Bus Selector16 : BusSelector  OutputSignals=lineSegValid,lineSegWidth,lineC0,lineC1,lineC2,lineC3,lineSegStart,lineSegEnd
  Bus Selector17 : BusSelector  OutputSignals=lineSegValid,lineSegWidth,lineC0,lineC1,lineC2,lineC3,lineSegStart,lineSegEnd
  Constant3 : Constant
  Constant5 : Constant
  Gain2 : Gain  OutDataTypeStr=single
  Gain3 : Gain  OutDataTypeStr=single
  LineInfo_Process : Subsystem  Commented=on
  LineInfo_Process1 : Subsystem
  LineInfo_Process2 : Subsystem
  Selector1 : Selector  IndexMode=Zero-based  InputPortWidth=4  IndexOptions=Index vector (dialog)  Indices=[0]  OutputSizes=1
  Selector13 : Selector  InputPortWidth=3  IndexOptions=Index vector (port)  Indices=[0]  OutputSizes=1
  Selector18 : Selector  InputPortWidth=3  IndexOptions=Index vector (port)  Indices=[0]  OutputSizes=1
  Selector4 : Selector  IndexMode=Zero-based  InputPortWidth=4  IndexOptions=Index vector (dialog)  Indices=[1]  OutputSizes=1
  Signal Conversion{,1..15} (16 alike) : SignalConversion  OverrideOpt=off
  LeftLineCoef : Outport
  RightLineCoef : Outport  Port=2
  wires:
  lineData/1 -> Selector4/1, Selector1/1
  Bus Selector14/1 -> Selector18/1  "<lineSegs>"
  Bus Selector16/1 -> Gain2/1  "<lineSegValid>"
  Bus Selector16/2 -> Signal Conversion1/1  "<lineSegWidth>"
  Bus Selector16/3 -> Signal Conversion2/1  "<lineC0>"
  Bus Selector16/4 -> Signal Conversion3/1  "<lineC1>"
  Bus Selector16/5 -> Signal Conversion4/1  "<lineC2>"
  Bus Selector16/6 -> Signal Conversion5/1  "<lineC3>"
  Gain2/1 -> Signal Conversion/1
  [Left_flg] -> Bus Creator3/1  "Valid"
  Constant3/1 -> Bus Creator3/10  "ValidDist"
  Signal Conversion/1 -> Bus Creator3/2  "LineQuality"
  Signal Conversion1/1 -> Bus Creator3/3  "LineWidth"
  Signal Conversion2/1 -> Bus Creator3/4  "LineA0"
  Signal Conversion3/1 -> Bus Creator3/5  "LineA1"
  Signal Conversion4/1 -> Bus Creator3/6  "LineA2"
  Signal Conversion5/1 -> Bus Creator3/7  "LineA3"
  Bus Selector16/7 -> Signal Conversion6/1  "<lineSegStart>"
  Bus Selector16/8 -> Signal Conversion7/1  "<lineSegEnd>"
  Signal Conversion6/1 -> Bus Creator3/8  "LineStart"
  Signal Conversion7/1 -> Bus Creator3/9  "LineEnd"
  Signal Conversion15/1 -> Bus Creator2/9  "LineEnd"
  Signal Conversion14/1 -> Bus Creator2/8  "LineStart"
  Bus Selector17/8 -> Signal Conversion15/1  "<lineSegEnd>"
  Bus Selector17/7 -> Signal Conversion14/1  "<lineSegStart>"
  Signal Conversion13/1 -> Bus Creator2/7  "LineA3"
  Signal Conversion12/1 -> Bus Creator2/6  "LineA2"
  Signal Conversion11/1 -> Bus Creator2/5  "LineA1"
  Signal Conversion10/1 -> Bus Creator2/4  "LineA0"
  Signal Conversion9/1 -> Bus Creator2/3  "LineWidth"
  Signal Conversion8/1 -> Bus Creator2/2  "LineQuality"
  Constant5/1 -> Bus Creator2/10  "ValidDist"
  [Right_flg] -> Bus Creator2/1  "Valid"
  Gain3/1 -> Signal Conversion8/1
  Bus Selector17/6 -> Signal Conversion13/1  "<lineC3>"
  Bus Selector17/5 -> Signal Conversion12/1  "<lineC2>"
  Bus Selector17/4 -> Signal Conversion11/1  "<lineC1>"
  Bus Selector17/3 -> Signal Conversion10/1  "<lineC0>"
  Bus Selector17/2 -> Signal Conversion9/1  "<lineSegWidth>"
  Bus Selector17/1 -> Gain3/1  "<lineSegValid>"
  Bus Selector15/1 -> Selector13/1  "<lineSegs>"
  Selector13/1 -> Bus Selector17/1
  Selector18/1 -> Bus Selector16/1
  Bus Creator3/1 -> LeftLineCoef/1
  Bus Creator2/1 -> RightLineCoef/1
  Selector1/1 -> LineInfo_Process1/1(Leftline_Data), Bus Selector14/1
  Selector4/1 -> LineInfo_Process2/1(Rightline_Data), Bus Selector15/1
  LineInfo_Process1/1(Index) -> Selector18/2
  LineInfo_Process1/2(Valid) -> [Left_flg]
  LineInfo_Process2/1(Index) -> Selector13/2
  LineInfo_Process2/2(Valid) -> [Right_flg]

== system TosAeb/ELK_Objsel/Select Object Info1 ==
  Objs_all : Inport
  Index : Inport  Port=2
  If : If  IfExpression=u1 == 255
  If Action Subsystem1 : Subsystem  TreatAsAtomicUnit=on
  If Action Subsystem2 : Subsystem  TreatAsAtomicUnit=on
  Merge : Merge
  TargetInfo : Outport  OutDataTypeStr=Bus: Object_Struct
  wires:
  [Index] -> If Action Subsystem1/1(Index), If/1
  If/1 -> If Action Subsystem2/Action
  If/2 -> If Action Subsystem1/Action
  If Action Subsystem2/1(Out) -> Merge/1
  If Action Subsystem1/1(TargetInfo) -> Merge/2
  Merge/1 -> TargetInfo/1
  Objs_all/1 -> [Objs_all]
  Index/1 -> [Index]
  [Objs_all] -> If Action Subsystem1/2(Objs_all)

== system TosAeb/ELK_Objsel/Select Object Info2 ==
  Objs_all : Inport
  Index : Inport  Port=2
  If : If  IfExpression=u1 == 255
  If Action Subsystem1 : Subsystem  TreatAsAtomicUnit=on
  If Action Subsystem2 : Subsystem  TreatAsAtomicUnit=on
  Merge : Merge
  TargetInfo : Outport  OutDataTypeStr=Bus: Object_Struct
  wires:
  [Objs_all] -> If Action Subsystem1/2(Objs_all)
  Index/1 -> [Index]
  Objs_all/1 -> [Objs_all]
  Merge/1 -> TargetInfo/1
  If Action Subsystem1/1(TargetInfo) -> Merge/2
  If Action Subsystem2/1(Out) -> Merge/1
  If/2 -> If Action Subsystem1/Action
  If/1 -> If Action Subsystem2/Action
  [Index] -> If/1, If Action Subsystem1/1(Index)

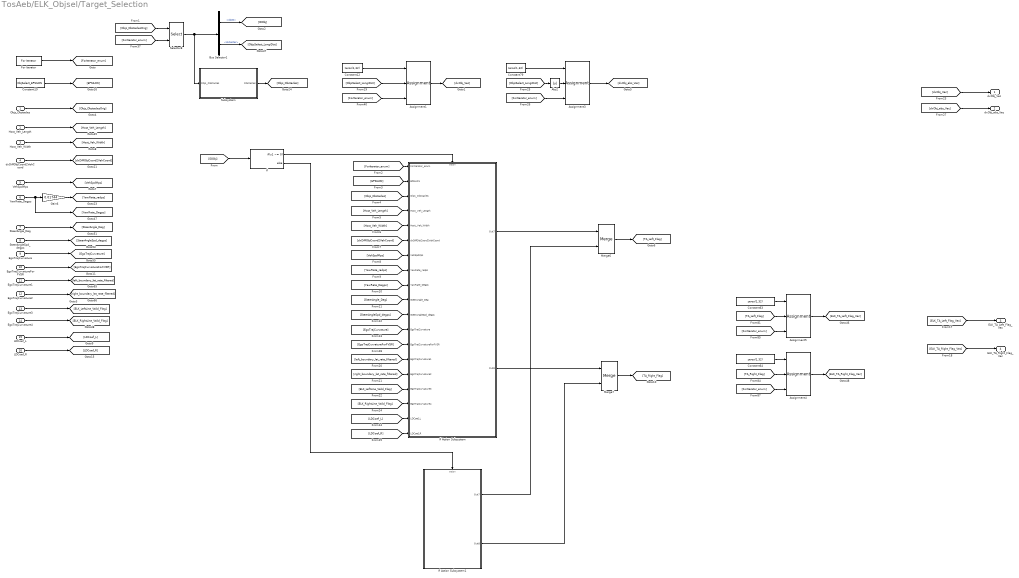
== system TosAeb/ELK_Objsel/Target_Selection ==
  Objs_Obstacles : Inport
  Host_Veh_Length : Inport  Port=2
  Host_Veh_Width : Inport  Port=3
  dxDiffObjCoord2VehCoord : Inport  Port=4
  VehSpdMps : Inport  Port=5
  YawRate_Degps : Inport  Port=6
  SteerAngle_Deg : Inport  Port=7
  SteerAngleSpd_degps : Inport  Port=8
  EgoTrajCurvature : Inport  Port=9
  EgoTrajCurvatureForFVSR : Inport  Port=10
  EgoTrajCurvature1 : Inport  Port=11
  EgoTrajCurvature2 : Inport  Port=12
  EgoTrajCurvature3 : Inport  Port=13
  EgoTrajCurvature4 : Inport  Port=14
  LDCoef_L : Inport  Port=15
  LDCoef_R : Inport  Port=16
  Abs2 : Abs
  Assignment{1,3..5} (4 alike) : Assignment  DiagnosticForDimensions=Warning  IndexOptions=Index vector (port)  Indices=1  OutputSizes=1
  Bus Selector1 : BusSelector  OutputSignals=objID,dxCenter
  Constant12 : Constant  Value=zeros(1,32)  OutDataTypeStr=single
  Constant19 : Constant  Value=ObjSelect_EPSILON
  Constant63 : Constant  Value=zeros(1,32)  OutDataTypeStr=boolean
  Constant64 : Constant  Value=zeros(1,32)  OutDataTypeStr=boolean
  Constant79 : Constant  Value=zeros(1,32)  OutDataTypeStr=single
  For Iterator : ForIterator  IterationLimit=32
  Gain6 : Gain  Gain=0.01744
  If : If  IfExpression=u1 ~= 0
  If Action Subsystem : Subsystem  TreatAsAtomicUnit=on
  If Action Subsystem1 : Subsystem  TreatAsAtomicUnit=on
  Merge6 : Merge
  Merge7 : Merge
  Selector8 : Selector  InputPortWidth=32  IndexOptions=Index vector (port)  Indices=1  OutputSizes=7
  Subsystem : Subsystem
  dxObj_Vec : Outport
  dxObj_abs_Vec : Outport  Port=2
  ELK_TA_Left_Flag_Vec : Outport  Port=3
  ELK_TA_Right_Flag_Vec : Outport  Port=4
  wires:
  Objs_Obstacles/1 -> [Objs_ObstaclesOrig]
  VehSpdMps/1 -> [VehSpdMps]
  Host_Veh_Width/1 -> [Host_Veh_Width]
  dxDiffObjCoord2VehCoord/1 -> [dxDiffObjCoord2VehCoord]
  YawRate_Degps/1 -> Gain6/1, [YawRate_Degps]
  Gain6/1 -> [YawRate_radps]
  Host_Veh_Length/1 -> [Host_Veh_Length]
  SteerAngle_Deg/1 -> [SteerAngle_Deg]
  SteerAngleSpd_degps/1 -> [SteerAngleSpd_degps]
  EgoTrajCurvature/1 -> [EgoTrajCurvature]
  LDCoef_L/1 -> [LDCoef_L]
  LDCoef_R/1 -> [LDCoef_R]
  Bus Selector1/2 -> [ObjsSelect_LongiDist]  "<dxCenter>"
  [Objs_ObstaclesOrig] -> Selector8/1
  [ForIterator_enum] -> Selector8/2
  Selector8/1 -> Bus Selector1/1, Subsystem/1(Objs_Obstacles)
  Assignment1/1 -> [dxObj_Vec]
  [ForIterator_enum] -> Assignment1/3
  Constant12/1 -> Assignment1/1
  [ObjsSelect_LongiDist] -> Assignment1/2
  [dxObj_Vec] -> dxObj_Vec/1
  Constant19/1 -> [EPSILON]
  Bus Selector1/1 -> [IDObj]  "<objID>"
  [IDObj] -> If/1
  If/1 -> If Action Subsystem/Action
  If/2 -> If Action Subsystem1/Action
  [ForIterator_enum] -> If Action Subsystem/1(ForIterator_enum)
  [EPSILON] -> If Action Subsystem/2(EPSILON)
  [Objs_Obstacles] -> If Action Subsystem/3(Objs_Obstacles)
  [Host_Veh_Length] -> If Action Subsystem/4(Host_Veh_Length)
  [Host_Veh_Width] -> If Action Subsystem/5(Host_Veh_Width)
  [dxDiffObjCoord2VehCoord] -> If Action Subsystem/6(dxDiffObjCoord2VehCoord)
  [VehSpdMps] -> If Action Subsystem/7(VehSpdMps)
  [YawRate_radps] -> If Action Subsystem/8(YawRate_radps)
  [YawRate_Degps] -> If Action Subsystem/9(YawRate_Degps)
  [SteerAngle_Deg] -> If Action Subsystem/10(SteerAngle_Deg)
  [SteerAngleSpd_degps] -> If Action Subsystem/11(SteerAngleSpd_degps)
  [EgoTrajCurvature] -> If Action Subsystem/12(EgoTrajCurvature)
  [LDCoef_L] -> If Action Subsystem/18(LDCoef_L)
  [LDCoef_R] -> If Action Subsystem/19(LDCoef_R)
  Assignment5/1 -> [ELK_TA_Left_Flag_Vec]
  [TA_Left_Flag] -> Assignment5/2
  [TA_Right_Flag] -> Assignment4/2
  Assignment4/1 -> [ELK_TA_Right_Flag_Vec]
  Constant63/1 -> Assignment5/1
  [ForIterator_enum] -> Assignment4/3
  [ForIterator_enum] -> Assignment5/3
  Constant64/1 -> Assignment4/1
  Merge6/1 -> [TA_Left_Flag]
  Merge7/1 -> [TA_Right_Flag]
  If Action Subsystem/1(Out7) -> Merge6/1
  If Action Subsystem/2(Out8) -> Merge7/1
  If Action Subsystem1/1(Out7) -> Merge6/2
  If Action Subsystem1/2(Out8) -> Merge7/2
  [ELK_TA_Left_Flag_Vec] -> ELK_TA_Left_Flag_Vec/1
  [ELK_TA_Right_Flag_Vec] -> ELK_TA_Right_Flag_Vec/1
  EgoTrajCurvature1/1 -> [left_boundary_lat_rate_filtered]
  EgoTrajCurvature2/1 -> [right_boundary_lat_rate_filtered]
  EgoTrajCurvature3/1 -> [ELK_LeftLine_Valid_Flag]
  EgoTrajCurvature4/1 -> [ELK_RightLine_Valid_Flag]
  [left_boundary_lat_rate_filtered] -> If Action Subsystem/14(EgoTrajCurvature1)
  [right_boundary_lat_rate_filtered] -> If Action Subsystem/15(EgoTrajCurvature2)
  [ELK_LeftLine_Valid_Flag] -> If Action Subsystem/16(EgoTrajCurvature3)
  [ELK_RightLine_Valid_Flag] -> If Action Subsystem/17(EgoTrajCurvature4)
  [ForIterator_enum] -> Assignment3/3
  [ObjsSelect_LongiDist] -> Abs2/1
  Assignment3/1 -> [dxObj_abs_Vec]
  Constant79/1 -> Assignment3/1
  Abs2/1 -> Assignment3/2
  [dxObj_abs_Vec] -> dxObj_abs_Vec/1
  EgoTrajCurvatureForFVSR/1 -> [EgoTrajCurvatureForFVSR]
  [EgoTrajCurvatureForFVSR] -> If Action Subsystem/13(EgoTrajCurvatureForFVSR)
  Subsystem/1(Obstacles) -> [Objs_Obstacles]
  For Iterator/1 -> [ForIterator_enum]
